simulink model "TosAeb"  (R2021a)
settings: Solver=FixedStepDiscrete  StartTime=0.0  StopTime=inf  FixedStep=0.01  RelTol=1e-3
undefined symbols (not defined in the file or in its folder): ACCModSts, AccSts1, AEB_ObjectMovingOrientation, AEB_ObjectMovingState, CN_BTCActive, CN_CurrentGearR, CN_CurrentGearR_DHT, CN_PTCActive, CN_VDCActive, CN_VSC_EspOff, CN_VSC_FCW_AEB_Error, CN_VSC_FCW_AEB_NoDispay, CN_VSC_FCW_AEB_PerfmDgdtn, CN_VSC_FCW_AEB_TempUnAvlbl, CN_VSC_VehStandstill, CNaccelAvoidMax, CNaccelAvoidMin, CNbrakePedalPress, CNcomfortStrLeftAccelMax, CNcomfortStrLeftAccelMin, CNcomfortStrRightAccelMax, CNcomfortStrRightAccelMin, CNDrvDoorOpen, CNDrvModeEco, CNDrvModeNorm, CNDrvModeOther, CNDrvModeSport, CNDrvSBRreminder, CNNull, CNobjInvalidID, CNPassDoorOpen, CNPassSBRreminder, CNPEBSAccelNegInf, CNPEBSAccelNull, CNPEBSTimeInf, CNPEBSTimeNull, CNSTOPMAX, CNSTOPNULL, CNsubjWidth, CNSwtOFF, CNSwtON, CNTTCMAX, CNTTCNULL, CNVehConfigAEBCycNone, CNVehConfigAEBNone, CNVehConfigAEBPedNone, CNVehConfigFCWNone, CNVehConfigJANone, CollisionSeverity, FALSE, FCWSnvty, JACycObjCalcInfoDefaultParams, JAPedObjCalcInfoDefaultParams, JAVehObjCalcInfoDefaultParams, latCycObjCalcInfoDefaultParams, latPedObjCalcInfoDefaultParams, longObjCalcInfoDefaultParams, ModSts, movingStatus, ObjectType ... (+1112)
libraries: simulink x3031, AEBSFunctionLib x50, Ta_Library x33, AEBSStateMachineLib x23, Mylibrary x12, simulink_extras x7, ObjSelect_lib x3, dspstat3 x2
(part 59 of 102)

== system TosAeb/AEB_Objsel/TA_Processor/PreProcessor/ObjectPrecessor/TAObjInfoCalc/For Each Subsystem/Info_Check1/BasicInfo_check/DyCorrect/Subsystem ==
  Obj_ID : Inport
  Compare To Constant : simulink/Logic and Bit Operations/Compare To Constant  relop=<=  const=0  OutDataTypeStr=boolean
  Constant : Constant  Value=P_TA_ObjAddAttrCalValue
  Constant1 : Constant  OutDataTypeStr=boolean
  Data Type Conversion : DataTypeConversion
  Logical Operator : Logic  Operator=OR  AllPortsSameDT=off  OutDataTypeStr=boolean
  Relational Operator : RelationalOperator  Operator=~=  OutDataTypeStr=boolean  RndMeth=Simplest
  Switch : Switch  Criteria=u2 > Threshold
  Unit Delay : UnitDelay  SampleTime=-1  HasFrameUpgradeWarning=on
  Obj_Invalid : Outport
  wires:
  Constant1/1 -> Switch/3
  Switch/1 -> Data Type Conversion/1
  Constant/1 -> Switch/2
  Logical Operator/1 -> Switch/1
  Relational Operator/1 -> Logical Operator/2
  Compare To Constant/1 -> Logical Operator/1
  Data Type Conversion/1 -> Obj_Invalid/1
  Unit Delay/1 -> Relational Operator/2
  Obj_ID/1 -> Unit Delay/1, Compare To Constant/1, Relational Operator/1

== system TosAeb/AEB_Objsel/TA_Processor/PreProcessor/ObjectPrecessor/TAObjInfoCalc/For Each Subsystem/Info_Check1/BasicInfo_check/DyCorrect/dy_charge ==
  Fus_ObjDy_Single : Inport
  Fus_ObsDyStd : Inport  Port=2
  Fus_ObjVy_Single : Inport  Port=3
  Veh_AEBFunState : Inport  Port=4
  Action Port : ActionPort
  Signal Conversion : SignalConversion  OverrideOpt=off
  Signal Conversion2 : SignalConversion  OverrideOpt=off
  Subsystem2 : Subsystem
  dy_change : Subsystem
  Fus_ObjDy : Outport
  Dy_CorrectFlg : Outport  Port=2
  wires:
  Signal Conversion2/1 -> Dy_CorrectFlg/1
  Signal Conversion/1 -> Fus_ObjDy/1
  dy_change/1(Fus_ObjDy) -> Signal Conversion/1
  Fus_ObsDyStd/1 -> Subsystem2/3(Fus_ObsDyStd)
  Veh_AEBFunState/1 -> Subsystem2/2(Veh_AEBFunState)
  Fus_ObjDy_Single/1 -> dy_change/2(Fus_ObjDy_Single), Subsystem2/4(Fus_ObjDy_Single)
  Fus_ObjVy_Single/1 -> Subsystem2/1(Fus_ObjVy_Single)
  Subsystem2/1(Dy_CorrectFlg) -> dy_change/1(Dy_CorrectFlg), Signal Conversion2/1

== system TosAeb/AEB_Objsel/TA_Processor/PreProcessor/ObjectPrecessor/TAObjInfoCalc/For Each Subsystem/Info_Check1/BasicInfo_check/DyCorrect/dy_nocharge ==
  Fus_ObjDy_Single : Inport
  Action Port : ActionPort
  Constant3 : Constant  Value=0  OutDataTypeStr=boolean
  Signal Conversion : SignalConversion  OverrideOpt=off
  Fus_ObjDy : Outport
  Dy_CorrectFlg : Outport  Port=2
  wires:
  Signal Conversion/1 -> Fus_ObjDy/1
  Constant3/1 -> Dy_CorrectFlg/1
  Fus_ObjDy_Single/1 -> Signal Conversion/1

== system TosAeb/AEB_Objsel/TA_Processor/PreProcessor/ObjectPrecessor/TAObjInfoCalc/For Each Subsystem/Info_Check1/BasicInfo_check/HeadAngle/If Action Subsystem ==
  Action Port : ActionPort
  Constant : Constant  Value=0  OutDataTypeStr=single
  Fus_ObjHeadAngle : Outport
  wires:
  Constant/1 -> Fus_ObjHeadAngle/1

== system TosAeb/AEB_Objsel/TA_Processor/PreProcessor/ObjectPrecessor/TAObjInfoCalc/For Each Subsystem/Info_Check1/BasicInfo_check/HeadAngle/If Action Subsystem1 ==
  Fus_Type : Inport
  ObsAngle_s : Inport  Port=2
  ObsAngleSTD_s : Inport  Port=3
  Obj_VxCr : Inport  Port=4
  Obj_VyCr : Inport  Port=5
  Action Port : ActionPort
  HeadAngle_c : Subsystem  TreatAsAtomicUnit=on
  Type_class : Subsystem
  Fus_ObjHeadAngle : Outport
  wires:
  Fus_Type/1 -> Type_class/1(Fus_Type)
  Type_class/1(tyoe_enable) -> HeadAngle_c/1(ID_Switch)
  Obj_VyCr/1 -> HeadAngle_c/4(Obj_VxCr)
  Obj_VxCr/1 -> HeadAngle_c/5(Obj_VyCr)
  ObsAngle_s/1 -> HeadAngle_c/2(ObsAngle_s)
  ObsAngleSTD_s/1 -> HeadAngle_c/3(ObsAngleSTD_s)
  HeadAngle_c/1(Fus_ObjHeadAngle) -> Fus_ObjHeadAngle/1

== system TosAeb/AEB_Objsel/TA_Processor/PreProcessor/ObjectPrecessor/TAObjInfoCalc/For Each Subsystem/Info_Check1/BasicInfo_check/HeadAngle/Subsystem ==
  Obj_ID : Inport
  Compare To Constant : simulink/Logic and Bit Operations/Compare To Constant  relop=<=  const=0  OutDataTypeStr=boolean
  Constant : Constant  Value=P_TA_ObjAddAttrCalValue
  Constant1 : Constant  OutDataTypeStr=boolean
  Data Type Conversion : DataTypeConversion
  Logical Operator : Logic  Operator=OR  AllPortsSameDT=off  OutDataTypeStr=boolean
  Relational Operator : RelationalOperator  Operator=~=  OutDataTypeStr=boolean  RndMeth=Simplest
  Switch : Switch  Criteria=u2 > Threshold
  Unit Delay : UnitDelay  SampleTime=-1  HasFrameUpgradeWarning=on
  Obj_Invalid : Outport
  wires:
  Switch/1 -> Data Type Conversion/1
  Constant1/1 -> Switch/3
  Constant/1 -> Switch/2
  Logical Operator/1 -> Switch/1
  Relational Operator/1 -> Logical Operator/2
  Compare To Constant/1 -> Logical Operator/1
  Data Type Conversion/1 -> Obj_Invalid/1
  Unit Delay/1 -> Relational Operator/2
  Obj_ID/1 -> Unit Delay/1, Compare To Constant/1, Relational Operator/1

== system TosAeb/AEB_Objsel/TA_Processor/PreProcessor/ObjectPrecessor/TAObjInfoCalc/For Each Subsystem/Info_Check1/BasicInfo_check/QuaCorrect/Subsystem ==
  Obj_ID : Inport
  Compare To Constant : simulink/Logic and Bit Operations/Compare To Constant  relop=<=  const=0  OutDataTypeStr=boolean
  Constant : Constant  Value=P_TA_ObjAddAttrCalValue
  Constant1 : Constant  OutDataTypeStr=boolean
  Data Type Conversion : DataTypeConversion
  Logical Operator : Logic  Operator=OR  AllPortsSameDT=off  OutDataTypeStr=boolean
  Relational Operator : RelationalOperator  Operator=~=  OutDataTypeStr=boolean  RndMeth=Simplest
  Switch : Switch  Criteria=u2 > Threshold
  Unit Delay : UnitDelay  SampleTime=-1  HasFrameUpgradeWarning=on
  Obj_Invalid : Outport
  wires:
  Switch/1 -> Data Type Conversion/1
  Constant1/1 -> Switch/3
  Constant/1 -> Switch/2
  Logical Operator/1 -> Switch/1
  Relational Operator/1 -> Logical Operator/2
  Compare To Constant/1 -> Logical Operator/1
  Data Type Conversion/1 -> Obj_Invalid/1
  Unit Delay/1 -> Relational Operator/2
  Obj_ID/1 -> Unit Delay/1, Compare To Constant/1, Relational Operator/1

== system TosAeb/AEB_Objsel/TA_Processor/PreProcessor/ObjectPrecessor/TAObjInfoCalc/For Each Subsystem/Info_Check1/BasicInfo_check/QuaCorrect/quality_recrectionelse ==
  Fus_TargetQuality : Inport
  Veh_AEBFunState : Inport  Port=2
  dy_flg : Inport  Port=3
  dx_flg : Inport  Port=4
  vx_flg : Inport  Port=5
  vy_flg : Inport  Port=6
  Action Port : ActionPort
  If : If
  Merge : Merge
  Quality_else : Subsystem
  Quality_if : Subsystem  TreatAsAtomicUnit=on
  Subsystem1 : Subsystem
  Fus_ObjQuality : Outport
  wires:
  dx_flg/1 -> Quality_else/3(dx_flg)
  dy_flg/1 -> Quality_else/2(dy_flg)
  vy_flg/1 -> Quality_else/5(vy_flg)
  vx_flg/1 -> Quality_else/4(vx_flg)
  Merge/1 -> Fus_ObjQuality/1
  Quality_else/1(Fus_ObjQuality) -> Merge/2
  Subsystem1/1(AEB_State) -> If/1
  If/2 -> Quality_else/Action
  If/1 -> Quality_if/Action
  Fus_TargetQuality/1 -> Quality_if/1(Fus_TargetQuality), Quality_else/1(Fus_TargetQuality)
  Veh_AEBFunState/1 -> Subsystem1/1(Veh_AEBFunState)
  Quality_if/1(Fus_ObjQuality) -> Merge/1

== system TosAeb/AEB_Objsel/TA_Processor/PreProcessor/ObjectPrecessor/TAObjInfoCalc/For Each Subsystem/Info_Check1/BasicInfo_check/QuaCorrect/quality_recrectionif ==
  Fus_TargetQuality : Inport
  Action Port : ActionPort
  Signal Conversion : SignalConversion  OverrideOpt=off
  Fus_ObjQuality : Outport
  wires:
  Signal Conversion/1 -> Fus_ObjQuality/1
  Fus_TargetQuality/1 -> Signal Conversion/1

== system TosAeb/AEB_Objsel/TA_Processor/PreProcessor/ObjectPrecessor/TAObjInfoCalc/For Each Subsystem/Info_Check1/BasicInfo_check/VxCorrect/If Action Subsystem ==
  Fus_ObjVx_Single : Inport
  Action Port : ActionPort
  Constant3 : Constant  Value=0  OutDataTypeStr=boolean
  Signal Conversion : SignalConversion  OverrideOpt=off
  Fus_ObjVx : Outport
  Vx_CorrectFlg : Outport  Port=2
  wires:
  Signal Conversion/1 -> Fus_ObjVx/1
  Constant3/1 -> Vx_CorrectFlg/1
  Fus_ObjVx_Single/1 -> Signal Conversion/1

== system TosAeb/AEB_Objsel/TA_Processor/PreProcessor/ObjectPrecessor/TAObjInfoCalc/For Each Subsystem/Info_Check1/BasicInfo_check/VxCorrect/Subsystem ==
  Obj_ID : Inport
  Compare To Constant : simulink/Logic and Bit Operations/Compare To Constant  relop=<=  const=0  OutDataTypeStr=boolean
  Constant : Constant  Value=P_TA_ObjAddAttrCalValue
  Constant1 : Constant  OutDataTypeStr=boolean
  Data Type Conversion : DataTypeConversion
  Logical Operator : Logic  Operator=OR  AllPortsSameDT=off  OutDataTypeStr=boolean
  Relational Operator : RelationalOperator  Operator=~=  OutDataTypeStr=boolean  RndMeth=Simplest
  Switch : Switch  Criteria=u2 > Threshold
  Unit Delay : UnitDelay  SampleTime=-1  HasFrameUpgradeWarning=on
  Obj_Invalid : Outport
  wires:
  Switch/1 -> Data Type Conversion/1
  Constant1/1 -> Switch/3
  Constant/1 -> Switch/2
  Logical Operator/1 -> Switch/1
  Relational Operator/1 -> Logical Operator/2
  Compare To Constant/1 -> Logical Operator/1
  Data Type Conversion/1 -> Obj_Invalid/1
  Unit Delay/1 -> Relational Operator/2
  Obj_ID/1 -> Unit Delay/1, Compare To Constant/1, Relational Operator/1

== system TosAeb/AEB_Objsel/TA_Processor/PreProcessor/ObjectPrecessor/TAObjInfoCalc/For Each Subsystem/Info_Check1/BasicInfo_check/VxCorrect/Subsystem3 ==
  Fus_ObjVx_Single : Inport
  Fus_ObjDx_Single : Inport  Port=2
  Fus_ObjVxStd : Inport  Port=3
  Veh_AEBFunState : Inport  Port=4
  Action Port : ActionPort
  Signal Conversion : SignalConversion  OverrideOpt=off
  Signal Conversion2 : SignalConversion  OverrideOpt=off
  Subsystem1 : Subsystem
  Subsystem2 : Subsystem
  Fus_ObjVx : Outport
  Vx_CorrectFlg : Outport  Port=2
  wires:
  Signal Conversion2/1 -> Vx_CorrectFlg/1
  Signal Conversion/1 -> Fus_ObjVx/1
  Subsystem1/1(Fus_ObjVx) -> Signal Conversion/1
  Veh_AEBFunState/1 -> Subsystem2/2(Veh_AEBFunState)
  Subsystem2/1(Vx_CorrectFlg) -> Signal Conversion2/1, Subsystem1/1(Vx_CorrectFlg)
  Fus_ObjDx_Single/1 -> Subsystem2/1(Fus_ObjDx_Single)
  Fus_ObjVx_Single/1 -> Subsystem1/2(Fus_ObjVx_Single), Subsystem2/4(Fus_ObjVx_Single)
  Fus_ObjVxStd/1 -> Subsystem2/3(Fus_ObjVxStd)

== system TosAeb/AEB_Objsel/TA_Processor/PreProcessor/ObjectPrecessor/TAObjInfoCalc/For Each Subsystem/Info_Check1/BasicInfo_check/VyCorrect/If Action Subsystem ==
  Fus_ObjVy_Single : Inport
  Action Port : ActionPort
  Constant3 : Constant  Value=0  OutDataTypeStr=boolean
  Signal Conversion : SignalConversion  OverrideOpt=off
  Fus_ObjVy : Outport
  Vy_CorrectFlg : Outport  Port=2
  wires:
  Signal Conversion/1 -> Fus_ObjVy/1
  Constant3/1 -> Vy_CorrectFlg/1
  Fus_ObjVy_Single/1 -> Signal Conversion/1

== system TosAeb/AEB_Objsel/TA_Processor/PreProcessor/ObjectPrecessor/TAObjInfoCalc/For Each Subsystem/Info_Check1/BasicInfo_check/VyCorrect/Subsystem ==
  Obj_ID : Inport
  Compare To Constant : simulink/Logic and Bit Operations/Compare To Constant  relop=<=  const=0  OutDataTypeStr=boolean
  Constant : Constant  Value=P_TA_ObjAddAttrCalValue
  Constant1 : Constant  OutDataTypeStr=boolean
  Data Type Conversion : DataTypeConversion
  Logical Operator : Logic  Operator=OR  AllPortsSameDT=off  OutDataTypeStr=boolean
  Relational Operator : RelationalOperator  Operator=~=  OutDataTypeStr=boolean  RndMeth=Simplest
  Switch : Switch  Criteria=u2 > Threshold
  Unit Delay : UnitDelay  SampleTime=-1  HasFrameUpgradeWarning=on
  Obj_Invalid : Outport
  wires:
  Switch/1 -> Data Type Conversion/1
  Constant1/1 -> Switch/3
  Constant/1 -> Switch/2
  Logical Operator/1 -> Switch/1
  Relational Operator/1 -> Logical Operator/2
  Compare To Constant/1 -> Logical Operator/1
  Data Type Conversion/1 -> Obj_Invalid/1
  Unit Delay/1 -> Relational Operator/2
  Obj_ID/1 -> Unit Delay/1, Compare To Constant/1, Relational Operator/1

== system TosAeb/AEB_Objsel/TA_Processor/PreProcessor/ObjectPrecessor/TAObjInfoCalc/For Each Subsystem/Info_Check1/BasicInfo_check/VyCorrect/Subsystem3 ==
  Fus_ObjVy_Single : Inport
  Fus_ObjDy_Single : Inport  Port=2
  Veh_AEBFunState : Inport  Port=3
  Fus_TargetState : Inport  Port=4
  Veh_YawRate : Inport  Port=5
  Fus_ObsVyStd : Inport  Port=6
  Action Port : ActionPort
  Signal Conversion : SignalConversion  OverrideOpt=off
  Signal Conversion2 : SignalConversion  OverrideOpt=off
  Subsystem1 : Subsystem
  Subsystem2 : Subsystem
  Fus_ObjVy : Outport
  Vy_CorrectFlg : Outport  Port=2
  wires:
  Subsystem2/2(State_flg) -> Subsystem1/2(State_flg)
  Fus_TargetState/1 -> Subsystem2/3(Fus_TargetState)
  Signal Conversion2/1 -> Vy_CorrectFlg/1
  Signal Conversion/1 -> Fus_ObjVy/1
  Fus_ObjVy_Single/1 -> Subsystem1/3(Fus_ObjVy_Single), Subsystem2/6(Fus_ObjVy_Single)
  Veh_AEBFunState/1 -> Subsystem2/2(Veh_AEBFunState)
  Subsystem2/1(Vy_CorrectFlg) -> Subsystem1/1(Vy_CorrectFlg), Signal Conversion2/1
  Fus_ObjDy_Single/1 -> Subsystem2/1(Fus_ObjDy_Single)
  Subsystem1/1(Fus_ObjVy) -> Signal Conversion/1
  Veh_YawRate/1 -> Subsystem2/5(Veh_YawRate)
  Fus_ObsVyStd/1 -> Subsystem2/4(Fus_ObsVyStd)

== system TosAeb/AEB_Objsel/TA_Processor/PreProcessor/ObjectPrecessor/TAObjInfoCalc/For Each Subsystem/Info_Check1/Basic_Info_check/AbsSpd/AbsVx_correct ==
  ObsVelXAbs_s : Inport
  Vhe_Spd : Inport  Port=2
  Obj_VxCr : Inport  Port=3
  Abs : Abs
  Add : Sum
  Add1 : Sum  Inputs=+-
  Compare To Constant : simulink/Logic and Bit Operations/Compare To Constant  relop=<=  const=2  OutDataTypeStr=boolean
  Switch : Switch  Criteria=u2 > Threshold
  Terminator : Terminator
  Fus_ObjVxAbs : Outport
  wires:
  Vhe_Spd/1 -> Add/1
  Obj_VxCr/1 -> Add/2
  Add/1 -> Switch/3, Add1/2
  ObsVelXAbs_s/1 -> Fus_ObjVxAbs/1, Switch/1, Add1/1
  Add1/1 -> Abs/1
  Compare To Constant/1 -> Switch/2
  Abs/1 -> Compare To Constant/1
  Switch/1 -> Terminator/1

== system TosAeb/AEB_Objsel/TA_Processor/PreProcessor/ObjectPrecessor/TAObjInfoCalc/For Each Subsystem/Info_Check1/Basic_Info_check/AbsSpd/AbsVy_correct ==
  ObsVelYAbs_s : Inport
  Veh_YawRate : Inport  Port=2
  Obj_VyCr : Inport  Port=3
  Abs : Abs
  Add : Sum
  Add1 : Sum  Inputs=+-  SaturateOnIntegerOverflow=on
  Compare To Constant : simulink/Logic and Bit Operations/Compare To Constant  relop=<=  const=1  OutDataTypeStr=boolean
  Constant : Constant  Value=P_TA_PrmForVehRearAxleDisttoFrnt
  Product : Product
  Switch : Switch  Criteria=u2 > Threshold
  Terminator : Terminator
  Fus_ObjVyAbs : Outport
  wires:
  Constant/1 -> Product/2
  Veh_YawRate/1 -> Product/1
  Product/1 -> Add/1
  Obj_VyCr/1 -> Add/2
  Add/1 -> Switch/3, Add1/2
  ObsVelYAbs_s/1 -> Fus_ObjVyAbs/1, Switch/1, Add1/1
  Add1/1 -> Abs/1
  Abs/1 -> Compare To Constant/1
  Compare To Constant/1 -> Switch/2
  Switch/1 -> Terminator/1

== system TosAeb/AEB_Objsel/TA_Processor/PreProcessor/ObjectPrecessor/TAObjInfoCalc/For Each Subsystem/Info_Check1/Basic_Info_check/AccCorrect/Acc_XY ==
  ObsAccY_s : Inport
  Obj_vy : Inport  Port=2
  ObsAccX_s : Inport  Port=3
  Obj_Vx : Inport  Port=4
  Veh_AccX : Inport  Port=5
  Veh_AccY : Inport  Port=6
  Veh_AEBSta : Inport  Port=7
  Obj_Id : Inport  Port=8
  Add : Sum  Inputs=+-
  Add1 : Sum  Inputs=+-
  Compare To Constant : simulink/Logic and Bit Operations/Compare To Constant  relop===  const=0  OutDataTypeStr=boolean
  Constant : Constant  Value=0  OutDataTypeStr=single
  Constant1 : Constant  Value=0  OutDataTypeStr=single
  Constant2 : Constant  Value=P_TA_ObjAccCalculateCalebration
  Constant3 : Constant  Value=P_TA_ObjAccCalculateCalebration
  Constant4 : Constant  Value=0.2  OutDataTypeStr=single
  LowPassFilter1 : Ta_Library/LowPassFilter  SystemSampleTime=-1  VariantActivationTime=update diagram  Latency=0  AutoFrameSizeCalculation=off
  Subsystem : Subsystem
  Subsystem1 : Subsystem
  Switch : Switch  Criteria=u2 > Threshold
  Switch1 : Switch  Criteria=u2 > Threshold
  Switch{2..5} (4 alike) : Switch  Criteria=u2 ~= 0
  Fus_ObjAy : Outport
  Fus_ObjAx : Outport  Port=2
  wires:
  Switch5/1 -> Switch3/1
  Constant3/1 -> Switch1/2
  Constant2/1 -> Switch/2
  Add1/1 -> Switch4/1, Subsystem1/1(ObsAccX_s), Switch1/1
  Add/1 -> Switch5/1, Subsystem/1(ObsAccY_s), Switch/1
  Obj_vy/1 -> Subsystem/2(Obj_vy)
  Subsystem/1(ObsAy_Correct) -> Switch/3
  Obj_Vx/1 -> Subsystem1/2(Obj_vX)
  Subsystem1/1(ObsAX_Correct) -> Switch1/3
  Veh_AEBSta/1 -> Compare To Constant/1
  Compare To Constant/1 -> [Aeb_state]
  ObsAccX_s/1 -> Add1/1
  Veh_AccX/1 -> Add1/2
  [Aeb_state] -> Switch2/2
  Constant/1 -> Switch2/3
  [Aeb_state] -> Switch3/2
  Constant1/1 -> Switch3/3
  ObsAccY_s/1 -> Add/1
  Switch3/1 -> Fus_ObjAy/1
  Veh_AccY/1 -> LowPassFilter1/2
  LowPassFilter1/1 -> Add/2
  Constant4/1 -> LowPassFilter1/1
  Switch2/1 -> Fus_ObjAx/1
  Obj_Id/1 -> Switch5/2, Switch4/2
  Switch1/1 -> Switch4/3
  Switch4/1 -> Switch2/1
  Switch/1 -> Switch5/3

== system TosAeb/AEB_Objsel/TA_Processor/PreProcessor/ObjectPrecessor/TAObjInfoCalc/For Each Subsystem/Info_Check1/Basic_Info_check/DxCorrect/Subsystem ==
  Obj_ID : Inport
  Compare To Constant : simulink/Logic and Bit Operations/Compare To Constant  relop=>  const=0  OutDataTypeStr=boolean
  Constant : Constant  Value=P_TA_ObjAddAttrCalValue
  Constant1 : Constant  OutDataTypeStr=boolean
  Data Type Conversion : DataTypeConversion
  Switch : Switch  Criteria=u2 > Threshold
  Obj_Invalid : Outport
  wires:
  Switch/1 -> Data Type Conversion/1
  Constant1/1 -> Switch/3
  Constant/1 -> Switch/2
  Compare To Constant/1 -> Switch/1
  Data Type Conversion/1 -> Obj_Invalid/1
  Obj_ID/1 -> Compare To Constant/1

== system TosAeb/AEB_Objsel/TA_Processor/PreProcessor/ObjectPrecessor/TAObjInfoCalc/For Each Subsystem/Info_Check1/Basic_Info_check/DxCorrect/dx_charge ==
  Fus_ObjDx_Single : Inport
  Fus_ObjDxStd : Inport  Port=2
  Fus_ObjVx_Single : Inport  Port=3
  Veh_AEBFunState : Inport  Port=4
  Action Port : ActionPort  ActionPortLabel=else
  Dx_Flag : Subsystem
  Signal Conversion : SignalConversion  OverrideOpt=off
  Signal Conversion2 : SignalConversion  OverrideOpt=off
  dx_change : Subsystem
  Fus_ObjDx : Outport
  Dx_CorrectFlg : Outport  Port=2
  wires:
  Signal Conversion2/1 -> Dx_CorrectFlg/1
  Signal Conversion/1 -> Fus_ObjDx/1
  Veh_AEBFunState/1 -> Dx_Flag/2(Veh_AEBFunState)
  Fus_ObjDx_Single/1 -> dx_change/2(Fus_ObjDx_Single), Dx_Flag/4(Fus_ObjDx_Single)
  Fus_ObjVx_Single/1 -> Dx_Flag/1(Fus_ObjVx_Single)
  dx_change/1(Fus_ObjDx) -> Signal Conversion/1
  Dx_Flag/1(Dx_CorrectFlg) -> dx_change/1(Dx_CorrectFlg), Signal Conversion2/1
  Fus_ObjDxStd/1 -> Dx_Flag/3(Fus_ObjDxStd)

== system TosAeb/AEB_Objsel/TA_Processor/PreProcessor/ObjectPrecessor/TAObjInfoCalc/For Each Subsystem/Info_Check1/Basic_Info_check/DxCorrect/dx_nocharge ==
  Fus_ObjDx_Single : Inport
  Action Port : ActionPort  ActionPortLabel=if(u1 > 0)
  Constant3 : Constant  Value=0  OutDataTypeStr=boolean
  Signal Conversion : SignalConversion  OverrideOpt=off
  Fus_ObjDx : Outport
  Dx_CorrectFlg : Outport  Port=2
  wires:
  Signal Conversion/1 -> Fus_ObjDx/1
  Constant3/1 -> Dx_CorrectFlg/1
  Fus_ObjDx_Single/1 -> Signal Conversion/1

== system TosAeb/AEB_Objsel/TA_Processor/PreProcessor/ObjectPrecessor/TAObjInfoCalc/For Each Subsystem/Info_Check1/Basic_Info_check/DxCorrect1/Subsystem ==
  Obj_ID : Inport
  Compare To Constant : simulink/Logic and Bit Operations/Compare To Constant  relop=<=  const=0  OutDataTypeStr=boolean
  Constant : Constant  Value=P_TA_ObjAddAttrCalValue
  Constant1 : Constant  OutDataTypeStr=boolean
  Data Type Conversion : DataTypeConversion
  Switch : Switch  Criteria=u2 > Threshold
  Obj_Invalid : Outport
  wires:
  Obj_ID/1 -> Compare To Constant/1
  Data Type Conversion/1 -> Obj_Invalid/1
  Compare To Constant/1 -> Switch/1
  Constant/1 -> Switch/2
  Constant1/1 -> Switch/3
  Switch/1 -> Data Type Conversion/1

== system TosAeb/AEB_Objsel/TA_Processor/PreProcessor/ObjectPrecessor/TAObjInfoCalc/For Each Subsystem/Info_Check1/Basic_Info_check/DxCorrect1/dx_charge ==
  Fus_ObjDx_Single : Inport
  Fus_ObjDxStd : Inport  Port=2
  Fus_ObjVx_Single : Inport  Port=3
  Veh_AEBFunState : Inport  Port=4
  Action Port : ActionPort
  Dx_Flag : Subsystem
  Signal Conversion : SignalConversion  OverrideOpt=off
  Signal Conversion2 : SignalConversion  OverrideOpt=off
  dx_change : Subsystem
  Fus_ObjDx : Outport
  Dx_CorrectFlg : Outport  Port=2
  wires:
  Fus_ObjDxStd/1 -> Dx_Flag/3(Fus_ObjDxStd)
  Dx_Flag/1(Dx_CorrectFlg) -> Signal Conversion2/1, dx_change/1(Dx_CorrectFlg)
  dx_change/1(Fus_ObjDx) -> Signal Conversion/1
  Fus_ObjVx_Single/1 -> Dx_Flag/1(Fus_ObjVx_Single)
  Fus_ObjDx_Single/1 -> Dx_Flag/4(Fus_ObjDx_Single), dx_change/2(Fus_ObjDx_Single)
  Veh_AEBFunState/1 -> Dx_Flag/2(Veh_AEBFunState)
  Signal Conversion/1 -> Fus_ObjDx/1
  Signal Conversion2/1 -> Dx_CorrectFlg/1

== system TosAeb/AEB_Objsel/TA_Processor/PreProcessor/ObjectPrecessor/TAObjInfoCalc/For Each Subsystem/Info_Check1/Basic_Info_check/DxCorrect1/dx_nocharge ==
  Fus_ObjDx_Single : Inport
  Action Port : ActionPort
  Constant3 : Constant  Value=0  OutDataTypeStr=boolean
  Signal Conversion : SignalConversion  OverrideOpt=off
  Fus_ObjDx : Outport
  Dx_CorrectFlg : Outport  Port=2
  wires:
  Fus_ObjDx_Single/1 -> Signal Conversion/1
  Constant3/1 -> Dx_CorrectFlg/1
  Signal Conversion/1 -> Fus_ObjDx/1

== system TosAeb/AEB_Objsel/TA_Processor/PreProcessor/ObjectPrecessor/TAObjInfoCalc/For Each Subsystem/Info_Check1/Basic_Info_check/DyCorrect/Subsystem ==
  Obj_ID : Inport
  Compare To Constant : simulink/Logic and Bit Operations/Compare To Constant  relop=>  const=0  OutDataTypeStr=boolean
  Constant : Constant  Value=P_TA_ObjAddAttrCalValue
  Constant1 : Constant  OutDataTypeStr=boolean
  Data Type Conversion : DataTypeConversion
  Switch : Switch  Criteria=u2 > Threshold
  Obj_Invalid : Outport
  wires:
  Constant1/1 -> Switch/3
  Switch/1 -> Data Type Conversion/1
  Constant/1 -> Switch/2
  Compare To Constant/1 -> Switch/1
  Data Type Conversion/1 -> Obj_Invalid/1
  Obj_ID/1 -> Compare To Constant/1

== system TosAeb/AEB_Objsel/TA_Processor/PreProcessor/ObjectPrecessor/TAObjInfoCalc/For Each Subsystem/Info_Check1/Basic_Info_check/DyCorrect/dy_charge ==
  Fus_ObjDy_Single : Inport
  Fus_ObsDyStd : Inport  Port=2
  Fus_ObjVy_Single : Inport  Port=3
  Veh_AEBFunState : Inport  Port=4
  Action Port : ActionPort  ActionPortLabel=else
  Signal Conversion : SignalConversion  OverrideOpt=off
  Signal Conversion2 : SignalConversion  OverrideOpt=off
  Subsystem2 : Subsystem
  dy_change : Subsystem
  Fus_ObjDy : Outport
  Dy_CorrectFlg : Outport  Port=2
  wires:
  Signal Conversion2/1 -> Dy_CorrectFlg/1
  Signal Conversion/1 -> Fus_ObjDy/1
  dy_change/1(Fus_ObjDy) -> Signal Conversion/1
  Fus_ObsDyStd/1 -> Subsystem2/3(Fus_ObsDyStd)
  Veh_AEBFunState/1 -> Subsystem2/2(Veh_AEBFunState)
  Fus_ObjDy_Single/1 -> dy_change/2(Fus_ObjDy_Single), Subsystem2/4(Fus_ObjDy_Single)
  Fus_ObjVy_Single/1 -> Subsystem2/1(Fus_ObjVy_Single)
  Subsystem2/1(Dy_CorrectFlg) -> dy_change/1(Dy_CorrectFlg), Signal Conversion2/1

== system TosAeb/AEB_Objsel/TA_Processor/PreProcessor/ObjectPrecessor/TAObjInfoCalc/For Each Subsystem/Info_Check1/Basic_Info_check/DyCorrect/dy_nocharge ==
  Fus_ObjDy_Single : Inport
  Action Port : ActionPort  ActionPortLabel=if(u1 > 0)
  Constant3 : Constant  Value=0  OutDataTypeStr=boolean
  Signal Conversion : SignalConversion  OverrideOpt=off
  Fus_ObjDy : Outport
  Dy_CorrectFlg : Outport  Port=2
  wires:
  Signal Conversion/1 -> Fus_ObjDy/1
  Constant3/1 -> Dy_CorrectFlg/1
  Fus_ObjDy_Single/1 -> Signal Conversion/1

== system TosAeb/AEB_Objsel/TA_Processor/PreProcessor/ObjectPrecessor/TAObjInfoCalc/For Each Subsystem/Info_Check1/Basic_Info_check/HeadAngle/If Action Subsystem ==
  Action Port : ActionPort  ActionPortLabel=if(u1 > 0)
  Constant : Constant  Value=0  OutDataTypeStr=single
  Fus_ObjHeadAngle : Outport
  wires:
  Constant/1 -> Fus_ObjHeadAngle/1

== system TosAeb/AEB_Objsel/TA_Processor/PreProcessor/ObjectPrecessor/TAObjInfoCalc/For Each Subsystem/Info_Check1/Basic_Info_check/HeadAngle/If Action Subsystem1 ==
  Fus_Type : Inport
  ObsAngle_s : Inport  Port=2
  ObsAngleSTD_s : Inport  Port=3
  Obj_VxCr : Inport  Port=4
  Obj_VyCr : Inport  Port=5
  Action Port : ActionPort  ActionPortLabel=else
  HeadAngle_c : Subsystem  TreatAsAtomicUnit=on
  Type_class : Subsystem
  Fus_ObjHeadAngle : Outport
  wires:
  Fus_Type/1 -> Type_class/1(Fus_Type)
  Type_class/1(tyoe_enable) -> HeadAngle_c/1(ID_Switch)
  Obj_VyCr/1 -> HeadAngle_c/4(Obj_VxCr)
  Obj_VxCr/1 -> HeadAngle_c/5(Obj_VyCr)
  ObsAngle_s/1 -> HeadAngle_c/2(ObsAngle_s)
  ObsAngleSTD_s/1 -> HeadAngle_c/3(ObsAngleSTD_s)
  HeadAngle_c/1(Fus_ObjHeadAngle) -> Fus_ObjHeadAngle/1

== system TosAeb/AEB_Objsel/TA_Processor/PreProcessor/ObjectPrecessor/TAObjInfoCalc/For Each Subsystem/Info_Check1/Basic_Info_check/HeadAngle/Subsystem ==
  Obj_ID : Inport
  Compare To Constant : simulink/Logic and Bit Operations/Compare To Constant  relop=>  const=0  OutDataTypeStr=boolean
  Constant : Constant  Value=P_TA_ObjAddAttrCalValue
  Constant1 : Constant  OutDataTypeStr=boolean
  Data Type Conversion : DataTypeConversion
  Switch : Switch  Criteria=u2 > Threshold
  Obj_Invalid : Outport
  wires:
  Switch/1 -> Data Type Conversion/1
  Constant1/1 -> Switch/3
  Constant/1 -> Switch/2
  Compare To Constant/1 -> Switch/1
  Data Type Conversion/1 -> Obj_Invalid/1
  Obj_ID/1 -> Compare To Constant/1

== system TosAeb/AEB_Objsel/TA_Processor/PreProcessor/ObjectPrecessor/TAObjInfoCalc/For Each Subsystem/Info_Check1/Basic_Info_check/QuaCorrect/Subsystem ==
  Obj_ID : Inport
  Compare To Constant : simulink/Logic and Bit Operations/Compare To Constant  relop=>  const=0  OutDataTypeStr=boolean
  Constant : Constant  Value=P_TA_ObjAddAttrCalValue
  Constant1 : Constant  OutDataTypeStr=boolean
  Data Type Conversion : DataTypeConversion
  Switch : Switch  Criteria=u2 > Threshold
  Obj_Invalid : Outport
  wires:
  Switch/1 -> Data Type Conversion/1
  Constant1/1 -> Switch/3
  Constant/1 -> Switch/2
  Compare To Constant/1 -> Switch/1
  Data Type Conversion/1 -> Obj_Invalid/1
  Obj_ID/1 -> Compare To Constant/1

== system TosAeb/AEB_Objsel/TA_Processor/PreProcessor/ObjectPrecessor/TAObjInfoCalc/For Each Subsystem/Info_Check1/Basic_Info_check/QuaCorrect/quality_recrectionelse ==
  Fus_TargetQuality : Inport
  Veh_AEBFunState : Inport  Port=2
  dy_flg : Inport  Port=3
  dx_flg : Inport  Port=4
  vx_flg : Inport  Port=5
  vy_flg : Inport  Port=6
  Action Port : ActionPort  ActionPortLabel=else
  If : If
  Merge : Merge
  Quality_else : Subsystem
  Quality_if : Subsystem  TreatAsAtomicUnit=on
  Subsystem1 : Subsystem
  Fus_ObjQuality : Outport
  wires:
  dx_flg/1 -> Quality_else/3(dx_flg)
  dy_flg/1 -> Quality_else/2(dy_flg)
  vy_flg/1 -> Quality_else/5(vy_flg)
  vx_flg/1 -> Quality_else/4(vx_flg)
  Merge/1 -> Fus_ObjQuality/1
  Quality_else/1(Fus_ObjQuality) -> Merge/2
  Subsystem1/1(AEB_State) -> If/1
  If/2 -> Quality_else/Action
  If/1 -> Quality_if/Action
  Fus_TargetQuality/1 -> Quality_if/1(Fus_TargetQuality), Quality_else/1(Fus_TargetQuality)
  Veh_AEBFunState/1 -> Subsystem1/1(Veh_AEBFunState)
  Quality_if/1(Fus_ObjQuality) -> Merge/1

== system TosAeb/AEB_Objsel/TA_Processor/PreProcessor/ObjectPrecessor/TAObjInfoCalc/For Each Subsystem/Info_Check1/Basic_Info_check/QuaCorrect/quality_recrectionif ==
  Fus_TargetQuality : Inport
  Action Port : ActionPort  ActionPortLabel=if(u1 > 0)
  Signal Conversion : SignalConversion  OverrideOpt=off
  Fus_ObjQuality : Outport
  wires:
  Signal Conversion/1 -> Fus_ObjQuality/1
  Fus_TargetQuality/1 -> Signal Conversion/1

== system TosAeb/AEB_Objsel/TA_Processor/PreProcessor/ObjectPrecessor/TAObjInfoCalc/For Each Subsystem/Info_Check1/Basic_Info_check/VxCorrect/If Action Subsystem ==
  Fus_ObjVx_Single : Inport
  Action Port : ActionPort  ActionPortLabel=if(u1 > 0)
  Constant3 : Constant  Value=0  OutDataTypeStr=boolean
  Signal Conversion : SignalConversion  OverrideOpt=off
  Fus_ObjVx : Outport
  Vx_CorrectFlg : Outport  Port=2
  wires:
  Signal Conversion/1 -> Fus_ObjVx/1
  Constant3/1 -> Vx_CorrectFlg/1
  Fus_ObjVx_Single/1 -> Signal Conversion/1

== system TosAeb/AEB_Objsel/TA_Processor/PreProcessor/ObjectPrecessor/TAObjInfoCalc/For Each Subsystem/Info_Check1/Basic_Info_check/VxCorrect/Subsystem ==
  Obj_ID : Inport
  Compare To Constant : simulink/Logic and Bit Operations/Compare To Constant  relop=>  const=0  OutDataTypeStr=boolean
  Constant : Constant  Value=P_TA_ObjAddAttrCalValue
  Constant1 : Constant  OutDataTypeStr=boolean
  Data Type Conversion : DataTypeConversion
  Switch : Switch  Criteria=u2 > Threshold
  Obj_Invalid : Outport
  wires:
  Switch/1 -> Data Type Conversion/1
  Constant1/1 -> Switch/3
  Constant/1 -> Switch/2
  Compare To Constant/1 -> Switch/1
  Data Type Conversion/1 -> Obj_Invalid/1
  Obj_ID/1 -> Compare To Constant/1

== system TosAeb/AEB_Objsel/TA_Processor/PreProcessor/ObjectPrecessor/TAObjInfoCalc/For Each Subsystem/Info_Check1/Basic_Info_check/VxCorrect/Subsystem3 ==
  Fus_ObjVx_Single : Inport
  Fus_ObjDx_Single : Inport  Port=2
  Fus_ObjVxStd : Inport  Port=3
  Veh_AEBFunState : Inport  Port=4
  Action Port : ActionPort  ActionPortLabel=else
  Signal Conversion : SignalConversion  OverrideOpt=off
  Signal Conversion2 : SignalConversion  OverrideOpt=off
  Subsystem1 : Subsystem
  Subsystem2 : Subsystem
  Fus_ObjVx : Outport
  Vx_CorrectFlg : Outport  Port=2
  wires:
  Signal Conversion2/1 -> Vx_CorrectFlg/1
  Signal Conversion/1 -> Fus_ObjVx/1
  Subsystem1/1(Fus_ObjVx) -> Signal Conversion/1
  Veh_AEBFunState/1 -> Subsystem2/2(Veh_AEBFunState)
  Subsystem2/1(Vx_CorrectFlg) -> Signal Conversion2/1, Subsystem1/1(Vx_CorrectFlg)
  Fus_ObjDx_Single/1 -> Subsystem2/1(Fus_ObjDx_Single)
  Fus_ObjVx_Single/1 -> Subsystem1/2(Fus_ObjVx_Single), Subsystem2/4(Fus_ObjVx_Single)
  Fus_ObjVxStd/1 -> Subsystem2/3(Fus_ObjVxStd)

== system TosAeb/AEB_Objsel/TA_Processor/PreProcessor/ObjectPrecessor/TAObjInfoCalc/For Each Subsystem/Info_Check1/Basic_Info_check/VyCorrect/If Action Subsystem ==
  Fus_ObjVy_Single : Inport
  Action Port : ActionPort  ActionPortLabel=if(u1 > 0)
  Constant3 : Constant  Value=0  OutDataTypeStr=boolean
  Signal Conversion : SignalConversion  OverrideOpt=off
  Fus_ObjVy : Outport
  Vy_CorrectFlg : Outport  Port=2
  wires:
  Signal Conversion/1 -> Fus_ObjVy/1
  Constant3/1 -> Vy_CorrectFlg/1
  Fus_ObjVy_Single/1 -> Signal Conversion/1

== system TosAeb/AEB_Objsel/TA_Processor/PreProcessor/ObjectPrecessor/TAObjInfoCalc/For Each Subsystem/Info_Check1/Basic_Info_check/VyCorrect/Subsystem ==
  Obj_ID : Inport
  Compare To Constant : simulink/Logic and Bit Operations/Compare To Constant  relop=>  const=0  OutDataTypeStr=boolean
  Constant : Constant  Value=P_TA_ObjAddAttrCalValue
  Constant1 : Constant  OutDataTypeStr=boolean
  Data Type Conversion : DataTypeConversion
  Switch : Switch  Criteria=u2 > Threshold
  Obj_Invalid : Outport
  wires:
  Switch/1 -> Data Type Conversion/1
  Constant1/1 -> Switch/3
  Constant/1 -> Switch/2
  Compare To Constant/1 -> Switch/1
  Data Type Conversion/1 -> Obj_Invalid/1
  Obj_ID/1 -> Compare To Constant/1

== system TosAeb/AEB_Objsel/TA_Processor/PreProcessor/ObjectPrecessor/TAObjInfoCalc/For Each Subsystem/Info_Check1/Basic_Info_check/VyCorrect/Subsystem3 ==
  Fus_ObjVy_Single : Inport
  Fus_ObjDy_Single : Inport  Port=2
  Veh_AEBFunState : Inport  Port=3
  Fus_TargetState : Inport  Port=4
  Veh_YawRate : Inport  Port=5
  Fus_ObsVyStd : Inport  Port=6
  Action Port : ActionPort  ActionPortLabel=else
  Signal Conversion : SignalConversion  OverrideOpt=off
  Signal Conversion2 : SignalConversion  OverrideOpt=off
  Subsystem1 : Subsystem
  Subsystem2 : Subsystem
  Fus_ObjVy : Outport
  Vy_CorrectFlg : Outport  Port=2
  wires:
  Subsystem2/2(State_flg) -> Subsystem1/2(State_flg)
  Fus_TargetState/1 -> Subsystem2/3(Fus_TargetState)
  Signal Conversion2/1 -> Vy_CorrectFlg/1
  Signal Conversion/1 -> Fus_ObjVy/1
  Fus_ObjVy_Single/1 -> Subsystem1/3(Fus_ObjVy_Single), Subsystem2/6(Fus_ObjVy_Single)
  Veh_AEBFunState/1 -> Subsystem2/2(Veh_AEBFunState)
  Subsystem2/1(Vy_CorrectFlg) -> Signal Conversion2/1, Subsystem1/1(Vy_CorrectFlg)
  Fus_ObjDy_Single/1 -> Subsystem2/1(Fus_ObjDy_Single)
  Subsystem1/1(Fus_ObjVy) -> Signal Conversion/1
  Veh_YawRate/1 -> Subsystem2/5(Veh_YawRate)
  Fus_ObsVyStd/1 -> Subsystem2/4(Fus_ObsVyStd)

== system TosAeb/AEB_Objsel/TA_Processor/PreProcessor/ObjectPrecessor/TAObjInfoCalc/For Each Subsystem/Info_Check1/Type_Process/Heading/Obj_AbsInfo ==
  VehSelfData : Inport
  TA_ObjInfo : Inport  Port=2
  For_Number : Inport  Port=3
  MapToAngularInterval : Ta_Library/MapToAngularInterval  SystemSampleTime=-1  Latency=0  AutoFrameSizeCalculation=off
  Subsystem : Subsystem
  Subsystem1 : Subsystem
  HeadingAnlge : Outport
  Obj_AbsAccly : Outport  Port=2
  Obj_AbsSpdy : Outport  Port=3
  wires:
  Subsystem/4(MaxAngle) -> MapToAngularInterval/2
  TA_ObjInfo/1 -> Subsystem/1(TA_ObjInfo)
  VehSelfData/1 -> Subsystem/3(VehSelfData)
  Subsystem/3(InputAngle) -> MapToAngularInterval/1
  MapToAngularInterval/1 -> Subsystem1/3(MappedAngle), HeadingAnlge/1
  Subsystem/1(<TA_ObjAccel>) -> Subsystem1/1(TA_ObjAccel)
  Subsystem/2(Obj_AbsVy) -> Subsystem1/2(Obj_AbsVy)
  Subsystem1/1(Obj_AbsAccly) -> Obj_AbsAccly/1
  Subsystem1/2(Obj_AbsSpdy) -> Obj_AbsSpdy/1
  For_Number/1 -> Subsystem/2(For_Number)

== system TosAeb/FcwAebJa_Step_sys/ALG_AEBSystem/ALG_AEBController/AEB_FuncCondCalcApp/CycFunctionCondCalc/cycAEBfullSuppAborCond/cycAEBfullSuppCondCalc/cycAEBfullSituationRelSuppCondCalc/LatDistCond4fullBrakeCalc/LatDistCondCalc ==
  dy : Inport
  latDistThres : Inport  Port=2
  Abs : Abs
  Relational Operator : RelationalOperator  Operator=>  OutDataTypeStr=boolean  RndMeth=Simplest
  latDistCond4fullBrake : Outport
  wires:
  Relational Operator/1 -> latDistCond4fullBrake/1
  Abs/1 -> Relational Operator/1
  dy/1 -> Abs/1
  latDistThres/1 -> Relational Operator/2

== system TosAeb/FcwAebJa_Step_sys/ALG_AEBSystem/ALG_AEBController/AEB_FuncCondCalcApp/CycFunctionCondCalc/cycAEBfullSuppAborCond/cycAEBfullSuppCondCalc/cycAEBfullSituationRelSuppCondCalc/LatDistCond4fullBrakeCalc/LatDistThresCalc ==
  FcwHMISetting : Inport
  vy : Inport  Port=2
  LateralDistanceThresHMISettingEarly : Subsystem  TreatAsAtomicUnit=on
  LateralDistanceThresHMISettingLate : Subsystem  TreatAsAtomicUnit=on
  Merge : Merge
  Switch Case : SwitchCase  CaseConditions={[FCWSnvty.HMISettingEarly,FCWSnvty.HMISettingMiddle]}
  latDistThres : Outport
  wires:
  vy/1 -> LateralDistanceThresHMISettingLate/1(vy)
  [HMISettingLate] -> LateralDistanceThresHMISettingLate/Action
  Switch Case/2 -> [HMISettingLate]
  Merge/1 -> latDistThres/1
  LateralDistanceThresHMISettingLate/1(latDistThres) -> Merge/2
  LateralDistanceThresHMISettingEarly/1(latDistThres) -> Merge/1
  Switch Case/1 -> LateralDistanceThresHMISettingEarly/Action
  FcwHMISetting/1 -> Switch Case/1

== system TosAeb/FcwAebJa_Step_sys/ALG_AEBSystem/ALG_AEBController/AEB_FuncCondCalcApp/CycFunctionCondCalc/cycAEBfullSuppAborCond/cycAEBfullSuppCondCalc/cycAEBfullVehRelSuppCondCalc/brakeReleventSuppCond/brakeReleventSuppCond_ESP ==
  ESP_MasterCylindBrakePressValid : Inport
  ESP_MasterCylindBrakePress : Inport  Port=2
  Action Port : ActionPort  ActionPortLabel=if(u1)
  Compare To Constant11 : simulink/Logic and Bit Operations/Compare To Constant  relop===  const=TRUE  OutDataTypeStr=boolean
  Compare To Constant12 : simulink/Logic and Bit Operations/Compare To Constant  relop=>  const=P_AEB_Func_cycAEBfullMasterCylindBrkPressSupThres  OutDataTypeStr=boolean
  Logical Operator1 : Logic  AllPortsSameDT=off  OutDataTypeStr=boolean
  brakeReleventSuppCond : Outport
  wires:
  Logical Operator1/1 -> brakeReleventSuppCond/1
  Compare To Constant12/1 -> Logical Operator1/2
  Compare To Constant11/1 -> Logical Operator1/1
  ESP_MasterCylindBrakePressValid/1 -> Compare To Constant11/1
  ESP_MasterCylindBrakePress/1 -> Compare To Constant12/1

== system TosAeb/FcwAebJa_Step_sys/ALG_AEBSystem/ALG_AEBController/AEB_FuncCondCalcApp/CycFunctionCondCalc/cycAEBfullSuppAborCond/cycAEBfullSuppCondCalc/cycAEBfullVehRelSuppCondCalc/brakeReleventSuppCond/brakeReleventSuppCond_IBooster ==
  note: 0x0:Not applied
      | 0x1:Applied
  Action Port : ActionPort  ActionPortLabel=else
  Constant : Constant  Value=FALSE
  brakeReleventSuppCond : Outport
  wires:
  Constant/1 -> brakeReleventSuppCond/1  "brakeReleventSuppCond"

== system TosAeb/FcwAebJa_Step_sys/ALG_AEBSystem/ALG_AEBController/AEB_FuncCondCalcApp/CycFunctionCondCalc/cycAEBpartSuppAborCond/cycAEBpartSuppCondCalc/cycAEBpartSituationRelActiveCondCalc/LatDistCond4PartBrakeCalc/LatDistCondCalc ==
  dy : Inport
  latDistThres : Inport  Port=2
  Abs : Abs
  Relational Operator : RelationalOperator  Operator=<=  OutDataTypeStr=boolean  RndMeth=Simplest
  latDistCond4PartBrake : Outport
  wires:
  latDistThres/1 -> Relational Operator/2
  dy/1 -> Abs/1
  Abs/1 -> Relational Operator/1
  Relational Operator/1 -> latDistCond4PartBrake/1

== system TosAeb/FcwAebJa_Step_sys/ALG_AEBSystem/ALG_AEBController/AEB_FuncCondCalcApp/CycFunctionCondCalc/cycAEBpartSuppAborCond/cycAEBpartSuppCondCalc/cycAEBpartSituationRelActiveCondCalc/LatDistCond4PartBrakeCalc/LatDistThresCalc ==
  FcwHMISetting : Inport
  vyCorr : Inport  Port=2
  Abs : Abs
  LateralDistanceThresHMISettingEarly : Subsystem  TreatAsAtomicUnit=on
  LateralDistanceThresHMISettingLate : Subsystem  TreatAsAtomicUnit=on
  Merge : Merge
  Switch Case : SwitchCase  CaseConditions={[FCWSnvty.HMISettingEarly,FCWSnvty.HMISettingMiddle]}
  latDistThres : Outport
  wires:
  Abs/1 -> LateralDistanceThresHMISettingLate/1(vyCorr), LateralDistanceThresHMISettingEarly/1(vyCorr)
  FcwHMISetting/1 -> Switch Case/1
  Switch Case/1 -> LateralDistanceThresHMISettingEarly/Action
  LateralDistanceThresHMISettingEarly/1(latDistThres) -> Merge/1
  LateralDistanceThresHMISettingLate/1(latDistThres) -> Merge/2
  Merge/1 -> latDistThres/1
  Switch Case/2 -> [HMISettingLate]
  [HMISettingLate] -> LateralDistanceThresHMISettingLate/Action
  vyCorr/1 -> Abs/1

== system TosAeb/FcwAebJa_Step_sys/ALG_AEBSystem/ALG_AEBController/AEB_FuncCondCalcApp/CycFunctionCondCalc/cycAEBpartSuppAborCond/cycAEBpartSuppCondCalc/cycAEBpartVehRelActiveCondCalc/brakeReleventSuppCond/brakeReleventSuppCond_ESP ==
  ESP_MasterCylindBrakePressValid : Inport
  ESP_MasterCylindBrakePress : Inport  Port=2
  Action Port : ActionPort  ActionPortLabel=if(u1)
  Compare To Constant11 : simulink/Logic and Bit Operations/Compare To Constant  relop===  const=TRUE  OutDataTypeStr=boolean
  Compare To Constant12 : simulink/Logic and Bit Operations/Compare To Constant  relop=>  const=P_AEB_Func_cycAEBpartMasterCylindBrkPressSupThres  OutDataTypeStr=boolean
  Logical Operator1 : Logic  AllPortsSameDT=off  OutDataTypeStr=boolean
  brakeReleventSuppCond : Outport
  wires:
  ESP_MasterCylindBrakePress/1 -> Compare To Constant12/1
  Compare To Constant12/1 -> Logical Operator1/2
  Logical Operator1/1 -> brakeReleventSuppCond/1
  ESP_MasterCylindBrakePressValid/1 -> Compare To Constant11/1
  Compare To Constant11/1 -> Logical Operator1/1

== system TosAeb/FcwAebJa_Step_sys/ALG_AEBSystem/ALG_AEBController/AEB_FuncCondCalcApp/CycFunctionCondCalc/cycAEBpartSuppAborCond/cycAEBpartSuppCondCalc/cycAEBpartVehRelActiveCondCalc/brakeReleventSuppCond/brakeReleventSuppCond_IBooster ==
  note: 0x0:Not applied
      | 0x1:Applied
  IB_BrkPedalAppld : Inport
  Action Port : ActionPort  ActionPortLabel=else
  Compare To Constant3 : simulink/Logic and Bit Operations/Compare To Constant  relop===  const=TRUE  OutDataTypeStr=boolean
  brakeReleventSuppCond : Outport
  wires:
  IB_BrkPedalAppld/1 -> Compare To Constant3/1
  Compare To Constant3/1 -> brakeReleventSuppCond/1  "brakeReleventSuppCond"

== system TosAeb/FcwAebJa_Step_sys/ALG_AEBSystem/ALG_AEBController/AEB_FuncCondCalcApp/CycFunctionCondCalc/cycPrefillSuppAborCond/cycPrefillSuppCondCalc/cycPrefillVehRelSuppCondCalc/brakeReleventSuppCond/brakeReleventSuppCond_ESP ==
  ESP_MasterCylindBrakePressValid : Inport
  ESP_MasterCylindBrakePress : Inport  Port=2
  Action Port : ActionPort  ActionPortLabel=if(u1)
  Compare To Constant11 : simulink/Logic and Bit Operations/Compare To Constant  relop===  const=TRUE  OutDataTypeStr=boolean
  Compare To Constant12 : simulink/Logic and Bit Operations/Compare To Constant  relop=>  const=P_AEB_Func_cycPrefillMasterCylindBrkPressSupThres  OutDataTypeStr=boolean
  Logical Operator1 : Logic  AllPortsSameDT=off  OutDataTypeStr=boolean
  brakeReleventSuppCond : Outport
  wires:
  Compare To Constant11/1 -> Logical Operator1/1
  Compare To Constant12/1 -> Logical Operator1/2
  ESP_MasterCylindBrakePress/1 -> Compare To Constant12/1
  Logical Operator1/1 -> brakeReleventSuppCond/1
  ESP_MasterCylindBrakePressValid/1 -> Compare To Constant11/1

== system TosAeb/FcwAebJa_Step_sys/ALG_AEBSystem/ALG_AEBController/AEB_FuncCondCalcApp/CycFunctionCondCalc/cycPrefillSuppAborCond/cycPrefillSuppCondCalc/cycPrefillVehRelSuppCondCalc/brakeReleventSuppCond/brakeReleventSuppCond_IBooster ==
  note: 0x0:Not applied
      | 0x1:Applied
  IB_BrkPedalAppld : Inport
  Action Port : ActionPort  ActionPortLabel=else
  Compare To Constant3 : simulink/Logic and Bit Operations/Compare To Constant  relop===  const=TRUE  OutDataTypeStr=boolean
  brakeReleventSuppCond : Outport
  wires:
  IB_BrkPedalAppld/1 -> Compare To Constant3/1
  Compare To Constant3/1 -> brakeReleventSuppCond/1  "brakeReleventSuppCond"

== system TosAeb/FcwAebJa_Step_sys/ALG_AEBSystem/ALG_AEBController/AEB_FuncCondCalcApp/JaCycFunctionCondCalc/JaCycAEBfullSuppAborCond/JaCycAEBfullSuppCondCalc/JaCycAEBfullSuppVehCondCalc/brakeReleventSuppCond/brakeReleventSuppCond_ESP ==
  ESP_MasterCylindBrakePress : Inport
  Action Port : ActionPort  ActionPortLabel=if(u1)
  Compare To Constant12 : simulink/Logic and Bit Operations/Compare To Constant  relop=>  const=P_AEB_Func_JaCycAEBfullMasterCylindBrkPressSupThres  OutDataTypeStr=boolean
  Constant : Constant  Value=P_AEB_Func_JaCycAEBfullBrkCondAborDelayTime
  Constant1 : Constant  Value=Ts
  StateDelayAbortion1 : AEBSFunctionLib/StateDelayAbortion  SystemSampleTime=-1  VariantActivationTime=update diagram  Latency=0  AutoFrameSizeCalculation=off
  brakeReleventSuppCond : Outport
  wires:
  StateDelayAbortion1/1 -> brakeReleventSuppCond/1
  Constant1/1 -> StateDelayAbortion1/3
  Constant/1 -> StateDelayAbortion1/2
  Compare To Constant12/1 -> StateDelayAbortion1/1
  ESP_MasterCylindBrakePress/1 -> Compare To Constant12/1

== system TosAeb/FcwAebJa_Step_sys/ALG_AEBSystem/ALG_AEBController/AEB_FuncCondCalcApp/JaCycFunctionCondCalc/JaCycAEBfullSuppAborCond/JaCycAEBfullSuppCondCalc/JaCycAEBfullSuppVehCondCalc/brakeReleventSuppCond/brakeReleventSuppCond_IBooster ==
  note: 0x0:Not applied
      | 0x1:Applied
  Action Port : ActionPort  ActionPortLabel=else
  Constant : Constant  Value=FALSE
  brakeReleventSuppCond : Outport
  wires:
  Constant/1 -> brakeReleventSuppCond/1  "brakeReleventSuppCond"

== system TosAeb/FcwAebJa_Step_sys/ALG_AEBSystem/ALG_AEBController/AEB_FuncCondCalcApp/JaCycFunctionCondCalc/JaCycPrefillSuppAborCond/JaCycPrefillSuppCondCalc/JaCycPrefillSuppVehCondCalc/brakeReleventSuppCond/brakeReleventSuppCond_ESP ==
  BrakePedalStatus_G : Inport
  ESP_MasterCylindBrakePress : Inport  Port=2
  Action Port : ActionPort  ActionPortLabel=if(u1)
  Compare To Constant1 : simulink/Logic and Bit Operations/Compare To Constant  relop===  const=TRUE  OutDataTypeStr=boolean
  Compare To Constant12 : simulink/Logic and Bit Operations/Compare To Constant  relop=>  const=P_AEB_Func_JaCycPrefillMasterCylindBrkPressSupThres  OutDataTypeStr=boolean
  Constant : Constant  Value=P_AEB_Func_JaCycPrefillBrkCondAborDelayTime
  Constant1 : Constant  Value=Ts
  Logical Operator3 : Logic  Operator=OR  AllPortsSameDT=off  OutDataTypeStr=boolean
  StateDelayAbortion1 : AEBSFunctionLib/StateDelayAbortion  SystemSampleTime=-1  VariantActivationTime=update diagram  Latency=0  AutoFrameSizeCalculation=off
  brakeReleventSuppCond : Outport
  wires:
  Compare To Constant1/1 -> Logical Operator3/1
  Logical Operator3/1 -> brakeReleventSuppCond/1
  Compare To Constant12/1 -> StateDelayAbortion1/1
  ESP_MasterCylindBrakePress/1 -> Compare To Constant12/1
  BrakePedalStatus_G/1 -> Compare To Constant1/1
  Constant/1 -> StateDelayAbortion1/2
  Constant1/1 -> StateDelayAbortion1/3
  StateDelayAbortion1/1 -> Logical Operator3/2

== system TosAeb/FcwAebJa_Step_sys/ALG_AEBSystem/ALG_AEBController/AEB_FuncCondCalcApp/JaCycFunctionCondCalc/JaCycPrefillSuppAborCond/JaCycPrefillSuppCondCalc/JaCycPrefillSuppVehCondCalc/brakeReleventSuppCond/brakeReleventSuppCond_IBooster ==
  note: 0x0:Not applied
      | 0x1:Applied
  IB_BrkPedalAppld : Inport
  Action Port : ActionPort  ActionPortLabel=else
  Compare To Constant3 : simulink/Logic and Bit Operations/Compare To Constant  relop===  const=TRUE  OutDataTypeStr=boolean
  brakeReleventSuppCond : Outport
  wires:
  Compare To Constant3/1 -> brakeReleventSuppCond/1  "brakeReleventSuppCond"
  IB_BrkPedalAppld/1 -> Compare To Constant3/1

== system TosAeb/FcwAebJa_Step_sys/ALG_AEBSystem/ALG_AEBController/AEB_FuncCondCalcApp/JaPedFunctionCondCalc/AEBJAPedAEBfullSuppAborCond/AEBJAPedAEBfullSuppCondCalc/AEBJAPedAEBfullSuppVehCondCalc/brakeReleventSuppCond/brakeReleventSuppCond_ESP ==
  ESP_MasterCylindBrakePress : Inport
  Action Port : ActionPort  ActionPortLabel=if(u1)
  Compare To Constant12 : simulink/Logic and Bit Operations/Compare To Constant  relop=>  const=P_AEB_Func_JaPedAEBfullMasterCylindBrkPressSupThres  OutDataTypeStr=boolean
  Constant : Constant  Value=P_AEB_Func_JaPedAEBfullBrkCondAborDelayTime
  Constant1 : Constant  Value=Ts
  StateDelayAbortion1 : AEBSFunctionLib/StateDelayAbortion  SystemSampleTime=-1  VariantActivationTime=update diagram  Latency=0  AutoFrameSizeCalculation=off
  brakeReleventSuppCond : Outport
  wires:
  ESP_MasterCylindBrakePress/1 -> Compare To Constant12/1
  Compare To Constant12/1 -> StateDelayAbortion1/1
  Constant/1 -> StateDelayAbortion1/2
  Constant1/1 -> StateDelayAbortion1/3
  StateDelayAbortion1/1 -> brakeReleventSuppCond/1

== system TosAeb/FcwAebJa_Step_sys/ALG_AEBSystem/ALG_AEBController/AEB_FuncCondCalcApp/JaPedFunctionCondCalc/AEBJAPedAEBfullSuppAborCond/AEBJAPedAEBfullSuppCondCalc/AEBJAPedAEBfullSuppVehCondCalc/brakeReleventSuppCond/brakeReleventSuppCond_IBooster ==
  note: 0x0:Not applied
      | 0x1:Applied
  Action Port : ActionPort  ActionPortLabel=else
  Constant : Constant  Value=FALSE
  brakeReleventSuppCond : Outport
  wires:
  Constant/1 -> brakeReleventSuppCond/1  "brakeReleventSuppCond"

== system TosAeb/FcwAebJa_Step_sys/ALG_AEBSystem/ALG_AEBController/AEB_FuncCondCalcApp/JaPedFunctionCondCalc/AEBJAPedPrefillSuppAborCond/AEBJAPedPrefillSuppCondCalc/JaPedPrefillSuppSubjCondCalcu/brakeReleventSuppCond/brakeReleventSuppCond_ESP ==
  BrakePedalStatus_G : Inport
  ESP_MasterCylindBrakePress : Inport  Port=2
  Action Port : ActionPort  ActionPortLabel=if(u1)
  Compare To Constant1 : simulink/Logic and Bit Operations/Compare To Constant  relop===  const=TRUE  OutDataTypeStr=boolean
  Compare To Constant12 : simulink/Logic and Bit Operations/Compare To Constant  relop=>  const=P_AEB_Func_JaPedPrefillMasterCylindBrkPressSupThres  OutDataTypeStr=boolean
  Constant : Constant  Value=P_AEB_Func_JaPedPrefillBrkCondAborDelayTime
  Constant1 : Constant  Value=Ts
  Logical Operator3 : Logic  Operator=OR  AllPortsSameDT=off  OutDataTypeStr=boolean
  StateDelayAbortion1 : AEBSFunctionLib/StateDelayAbortion  SystemSampleTime=-1  VariantActivationTime=update diagram  Latency=0  AutoFrameSizeCalculation=off
  brakeReleventSuppCond : Outport
  wires:
  StateDelayAbortion1/1 -> Logical Operator3/2
  Constant1/1 -> StateDelayAbortion1/3
  Constant/1 -> StateDelayAbortion1/2
  BrakePedalStatus_G/1 -> Compare To Constant1/1
  ESP_MasterCylindBrakePress/1 -> Compare To Constant12/1
  Compare To Constant12/1 -> StateDelayAbortion1/1
  Logical Operator3/1 -> brakeReleventSuppCond/1
  Compare To Constant1/1 -> Logical Operator3/1

== system TosAeb/FcwAebJa_Step_sys/ALG_AEBSystem/ALG_AEBController/AEB_FuncCondCalcApp/JaPedFunctionCondCalc/AEBJAPedPrefillSuppAborCond/AEBJAPedPrefillSuppCondCalc/JaPedPrefillSuppSubjCondCalcu/brakeReleventSuppCond/brakeReleventSuppCond_IBooster ==
  note: 0x0:Not applied
      | 0x1:Applied
  IB_BrkPedalAppld : Inport
  Action Port : ActionPort  ActionPortLabel=else
  Compare To Constant3 : simulink/Logic and Bit Operations/Compare To Constant  relop===  const=TRUE  OutDataTypeStr=boolean
  brakeReleventSuppCond : Outport
  wires:
  IB_BrkPedalAppld/1 -> Compare To Constant3/1
  Compare To Constant3/1 -> brakeReleventSuppCond/1  "brakeReleventSuppCond"
